simulink model "chapter2_5"  (R2022a)
settings: Solver=FixedStepAuto  StartTime=0.0  StopTime=8  FixedStep=3e-3  RelTol=1e-3
values from neighbouring files (symbols this model uses):
  A = 10    [Figure2_3.m]    (also in parameterLoadForchapter2_4.m = 0.0001; parameterLoadForchapter2_5.m = 0.0001)
  base = Mb*9.8/4+mw*9.8+0.5*9.8*Mv    (= 46305)    [parameterLoadForchapter2_5.m]
  d = 2.6    [parameterLoadForchapter2_4.m]    (also in parameterLoadForchapter2_5.m)
  I = 3055e-8    (= 3.055e-05)    [parameterLoadForchapter2_5.m]
  Jv = 3044    [parameterLoadForchapter2_4.m]    (also in parameterLoadForchapter2_5.m)
  kf = 6.67e7    (= 66700000)    [parameterLoadForchapter2_5.m]
  Kv = 1.5e6    (= 1500000)    [parameterLoadForchapter2_4.m]    (also in parameterLoadForchapter2_5.m)
  L = 15.6    [parameterLoadForchapter2_4.m]    (also in parameterLoadForchapter2_5.m = 210)
  Ln = LNs(i)    [Mchapter2_4.m]    (also in Mchapter2_5.m = LN_set(i))
  m = 7800*(7684e-6)    (= 59.9352)    [parameterLoadForchapter2_5.m]
  Mv = 4060    [parameterLoadForchapter2_4.m]    (also in parameterLoadForchapter2_5.m = 2030)
  mw = 1670    [parameterLoadForchapter2_4.m]    (also in parameterLoadForchapter2_5.m = 835)
  N1 = 0    [Mchapter2_5.m]
  N2 = 0    [Mchapter2_5.m]
  position = 0.5*L    (= 105)    [parameterLoadForchapter2_5.m]
  r = 0.42    [parameterLoadForchapter2_4.m]    (also in parameterLoadForchapter2_5.m)
  R = 400    [Figure2_3.m]    (also in parameterLoadForchapter2_4.m = 0.42; parameterLoadForchapter2_5.m = 0.42)
  v = vkmh/3.6    (= 13.8888888889)    [parameterLoadForchapter2_4.m]    (also in parameterLoadForchapter2_5.m = 27.7777777778)
  vkmh = 50    [parameterLoadForchapter2_4.m]    (also in parameterLoadForchapter2_5.m = 100)
(part 26 of 157)

== system chapter2_5/Subsystem5/Subsystem3/Subsystem2/Subsystem2 ==
  index : Inport
  F : Inport  Port=2
  Add : Sum
  Constant1 : Constant  Value=2
  Constant3 : Constant
  Demux : Demux  Outputs=2
  Subsystem : Subsystem
  Subsystem1 : Subsystem
  Subsystem2 : Subsystem
  Subsystem3 : Subsystem
  Out1 : Outport
  wires:
  Add/1 -> Out1/1
  Constant1/1 -> Subsystem1/2(k)
  Subsystem1/1(Out1) -> Subsystem2/1(uxt1)
  Subsystem1/2(tk) -> Subsystem2/2(tk)
  Subsystem/2(tk) -> Subsystem3/2(tk)
  Constant3/1 -> Subsystem/2(k)
  Subsystem/1(Out1) -> Subsystem3/1(uxt1)
  index/1 -> Subsystem/1(In1), Subsystem1/1(index)
  Subsystem3/1(U1) -> Add/1
  Subsystem2/1(U1) -> Add/2
  F/1 -> Demux/1
  Demux/1 -> Subsystem/3(In3)
  Demux/2 -> Subsystem1/3(F)

== system chapter2_5/Subsystem5/Subsystem3/Subsystem2/Subsystem21 ==
  index : Inport
  F : Inport  Port=2
  Add : Sum
  Constant1 : Constant  Value=2
  Constant3 : Constant
  Demux : Demux  Outputs=2
  Subsystem : Subsystem
  Subsystem1 : Subsystem
  Subsystem2 : Subsystem
  Subsystem3 : Subsystem
  Out1 : Outport
  wires:
  Add/1 -> Out1/1
  Constant1/1 -> Subsystem1/2(k)
  Subsystem1/1(Out1) -> Subsystem2/1(uxt1)
  Subsystem1/2(tk) -> Subsystem2/2(tk)
  Subsystem/2(tk) -> Subsystem3/2(tk)
  Constant3/1 -> Subsystem/2(k)
  Subsystem/1(Out1) -> Subsystem3/1(uxt1)
  index/1 -> Subsystem/1(In1), Subsystem1/1(index)
  Subsystem3/1(U1) -> Add/1
  Subsystem2/1(U1) -> Add/2
  F/1 -> Demux/1
  Demux/1 -> Subsystem/3(In3)
  Demux/2 -> Subsystem1/3(F)

== system chapter2_5/Subsystem5/Subsystem3/Subsystem2/Subsystem3 ==
  index : Inport
  F : Inport  Port=2
  Add : Sum
  Constant1 : Constant  Value=2
  Constant3 : Constant
  Demux : Demux  Outputs=2
  Subsystem : Subsystem
  Subsystem1 : Subsystem
  Subsystem2 : Subsystem
  Subsystem3 : Subsystem
  Out1 : Outport
  wires:
  Demux/2 -> Subsystem1/3(F)
  Demux/1 -> Subsystem/3(In3)
  F/1 -> Demux/1
  Subsystem2/1(U1) -> Add/2
  Subsystem3/1(U1) -> Add/1
  index/1 -> Subsystem1/1(index), Subsystem/1(In1)
  Subsystem/1(Out1) -> Subsystem3/1(uxt1)
  Constant3/1 -> Subsystem/2(k)
  Subsystem/2(tk) -> Subsystem3/2(tk)
  Subsystem1/2(tk) -> Subsystem2/2(tk)
  Subsystem1/1(Out1) -> Subsystem2/1(uxt1)
  Constant1/1 -> Subsystem1/2(k)
  Add/1 -> Out1/1

== system chapter2_5/Subsystem5/Subsystem3/Subsystem2/Subsystem4 ==
  index : Inport
  F : Inport  Port=2
  Add : Sum
  Constant1 : Constant  Value=2
  Constant3 : Constant
  Demux : Demux  Outputs=2
  Subsystem : Subsystem
  Subsystem1 : Subsystem
  Subsystem2 : Subsystem
  Subsystem3 : Subsystem
  Out1 : Outport
  wires:
  Add/1 -> Out1/1
  Constant1/1 -> Subsystem1/2(k)
  Subsystem1/1(Out1) -> Subsystem2/1(uxt1)
  Subsystem1/2(tk) -> Subsystem2/2(tk)
  Subsystem/2(tk) -> Subsystem3/2(tk)
  Constant3/1 -> Subsystem/2(k)
  Subsystem/1(Out1) -> Subsystem3/1(uxt1)
  index/1 -> Subsystem/1(In1), Subsystem1/1(index)
  Subsystem3/1(U1) -> Add/1
  Subsystem2/1(U1) -> Add/2
  F/1 -> Demux/1
  Demux/1 -> Subsystem/3(In3)
  Demux/2 -> Subsystem1/3(F)

== system chapter2_5/Subsystem5/Subsystem3/Subsystem2/Subsystem5 ==
  index : Inport
  F : Inport  Port=2
  Add : Sum
  Constant1 : Constant  Value=2
  Constant3 : Constant
  Demux : Demux  Outputs=2
  Subsystem : Subsystem
  Subsystem1 : Subsystem
  Subsystem2 : Subsystem
  Subsystem3 : Subsystem
  Out1 : Outport
  wires:
  Demux/2 -> Subsystem1/3(F)
  Demux/1 -> Subsystem/3(In3)
  F/1 -> Demux/1
  Subsystem2/1(U1) -> Add/2
  Subsystem3/1(U1) -> Add/1
  index/1 -> Subsystem1/1(index), Subsystem/1(In1)
  Subsystem/1(Out1) -> Subsystem3/1(uxt1)
  Constant3/1 -> Subsystem/2(k)
  Subsystem/2(tk) -> Subsystem3/2(tk)
  Subsystem1/2(tk) -> Subsystem2/2(tk)
  Subsystem1/1(Out1) -> Subsystem2/1(uxt1)
  Constant1/1 -> Subsystem1/2(k)
  Add/1 -> Out1/1

== system chapter2_5/Subsystem5/Subsystem3/Subsystem2/Subsystem6 ==
  index : Inport
  F : Inport  Port=2
  Add : Sum
  Constant1 : Constant  Value=2
  Constant3 : Constant
  Demux : Demux  Outputs=2
  Subsystem : Subsystem
  Subsystem1 : Subsystem
  Subsystem2 : Subsystem
  Subsystem3 : Subsystem
  Out1 : Outport
  wires:
  Demux/2 -> Subsystem1/3(F)
  Demux/1 -> Subsystem/3(In3)
  F/1 -> Demux/1
  Subsystem2/1(U1) -> Add/2
  Subsystem3/1(U1) -> Add/1
  index/1 -> Subsystem1/1(index), Subsystem/1(In1)
  Subsystem/1(Out1) -> Subsystem3/1(uxt1)
  Constant3/1 -> Subsystem/2(k)
  Subsystem/2(tk) -> Subsystem3/2(tk)
  Subsystem1/2(tk) -> Subsystem2/2(tk)
  Subsystem1/1(Out1) -> Subsystem2/1(uxt1)
  Constant1/1 -> Subsystem1/2(k)
  Add/1 -> Out1/1

== system chapter2_5/Subsystem5/Subsystem3/Subsystem2/Subsystem7 ==
  index : Inport
  F : Inport  Port=2
  Add : Sum
  Constant1 : Constant  Value=2
  Constant3 : Constant
  Demux : Demux  Outputs=2
  Subsystem : Subsystem
  Subsystem1 : Subsystem
  Subsystem2 : Subsystem
  Subsystem3 : Subsystem
  Out1 : Outport
  wires:
  Add/1 -> Out1/1
  Constant1/1 -> Subsystem1/2(k)
  Subsystem1/1(Out1) -> Subsystem2/1(uxt1)
  Subsystem1/2(tk) -> Subsystem2/2(tk)
  Subsystem/2(tk) -> Subsystem3/2(tk)
  Constant3/1 -> Subsystem/2(k)
  Subsystem/1(Out1) -> Subsystem3/1(uxt1)
  index/1 -> Subsystem/1(In1), Subsystem1/1(index)
  Subsystem3/1(U1) -> Add/1
  Subsystem2/1(U1) -> Add/2
  F/1 -> Demux/1
  Demux/1 -> Subsystem/3(In3)
  Demux/2 -> Subsystem1/3(F)

== system chapter2_5/Subsystem5/Subsystem3/Subsystem2/Subsystem8 ==
  index : Inport
  F : Inport  Port=2
  Add : Sum
  Constant1 : Constant  Value=2
  Constant3 : Constant
  Demux : Demux  Outputs=2
  Subsystem : Subsystem
  Subsystem1 : Subsystem
  Subsystem2 : Subsystem
  Subsystem3 : Subsystem
  Out1 : Outport
  wires:
  Add/1 -> Out1/1
  Constant1/1 -> Subsystem1/2(k)
  Subsystem1/1(Out1) -> Subsystem2/1(uxt1)
  Subsystem1/2(tk) -> Subsystem2/2(tk)
  Subsystem/2(tk) -> Subsystem3/2(tk)
  Constant3/1 -> Subsystem/2(k)
  Subsystem/1(Out1) -> Subsystem3/1(uxt1)
  index/1 -> Subsystem/1(In1), Subsystem1/1(index)
  Subsystem3/1(U1) -> Add/1
  Subsystem2/1(U1) -> Add/2
  F/1 -> Demux/1
  Demux/1 -> Subsystem/3(In3)
  Demux/2 -> Subsystem1/3(F)

== system chapter2_5/Subsystem5/Subsystem3/Subsystem2/Subsystem9 ==
  index : Inport
  F : Inport  Port=2
  Add : Sum
  Constant1 : Constant  Value=2
  Constant3 : Constant
  Demux : Demux  Outputs=2
  Subsystem : Subsystem
  Subsystem1 : Subsystem
  Subsystem2 : Subsystem
  Subsystem3 : Subsystem
  Out1 : Outport
  wires:
  Demux/2 -> Subsystem1/3(F)
  Demux/1 -> Subsystem/3(In3)
  F/1 -> Demux/1
  Subsystem2/1(U1) -> Add/2
  Subsystem3/1(U1) -> Add/1
  index/1 -> Subsystem1/1(index), Subsystem/1(In1)
  Subsystem/1(Out1) -> Subsystem3/1(uxt1)
  Constant3/1 -> Subsystem/2(k)
  Subsystem/2(tk) -> Subsystem3/2(tk)
  Subsystem1/2(tk) -> Subsystem2/2(tk)
  Subsystem1/1(Out1) -> Subsystem2/1(uxt1)
  Constant1/1 -> Subsystem1/2(k)
  Add/1 -> Out1/1

== system chapter2_5/Subsystem5/Subsystem3/Subsystem3/Subsystem1 ==
  index : Inport
  F : Inport  Port=2
  Add : Sum
  Constant1 : Constant  Value=2
  Constant3 : Constant
  Demux : Demux  Outputs=2
  Subsystem : Subsystem
  Subsystem1 : Subsystem
  Subsystem2 : Subsystem
  Subsystem3 : Subsystem
  Out1 : Outport
  wires:
  Add/1 -> Out1/1
  Constant1/1 -> Subsystem1/2(k)
  Subsystem1/1(Out1) -> Subsystem2/1(uxt1)
  Subsystem1/2(tk) -> Subsystem2/2(tk)
  Subsystem/2(tk) -> Subsystem3/2(tk)
  Constant3/1 -> Subsystem/2(k)
  Subsystem/1(Out1) -> Subsystem3/1(uxt1)
  index/1 -> Subsystem/1(In1), Subsystem1/1(index)
  Subsystem3/1(U1) -> Add/1
  Subsystem2/1(U1) -> Add/2
  F/1 -> Demux/1
  Demux/1 -> Subsystem/3(In3)
  Demux/2 -> Subsystem1/3(F)

== system chapter2_5/Subsystem5/Subsystem3/Subsystem3/Subsystem10 ==
  index : Inport
  F : Inport  Port=2
  Add : Sum
  Constant1 : Constant  Value=2
  Constant3 : Constant
  Demux : Demux  Outputs=2
  Subsystem : Subsystem
  Subsystem1 : Subsystem
  Subsystem2 : Subsystem
  Subsystem3 : Subsystem
  Out1 : Outport
  wires:
  Add/1 -> Out1/1
  Constant1/1 -> Subsystem1/2(k)
  Subsystem1/1(Out1) -> Subsystem2/1(uxt1)
  Subsystem1/2(tk) -> Subsystem2/2(tk)
  Subsystem/2(tk) -> Subsystem3/2(tk)
  Constant3/1 -> Subsystem/2(k)
  Subsystem/1(Out1) -> Subsystem3/1(uxt1)
  index/1 -> Subsystem/1(In1), Subsystem1/1(index)
  Subsystem3/1(U1) -> Add/1
  Subsystem2/1(U1) -> Add/2
  F/1 -> Demux/1
  Demux/1 -> Subsystem/3(In3)
  Demux/2 -> Subsystem1/3(F)

== system chapter2_5/Subsystem5/Subsystem3/Subsystem3/Subsystem11 ==
  index : Inport
  F : Inport  Port=2
  Add : Sum
  Constant1 : Constant  Value=2
  Constant3 : Constant
  Demux : Demux  Outputs=2
  Subsystem : Subsystem
  Subsystem1 : Subsystem
  Subsystem2 : Subsystem
  Subsystem3 : Subsystem
  Out1 : Outport
  wires:
  Demux/2 -> Subsystem1/3(F)
  Demux/1 -> Subsystem/3(In3)
  F/1 -> Demux/1
  Subsystem2/1(U1) -> Add/2
  Subsystem3/1(U1) -> Add/1
  index/1 -> Subsystem1/1(index), Subsystem/1(In1)
  Subsystem/1(Out1) -> Subsystem3/1(uxt1)
  Constant3/1 -> Subsystem/2(k)
  Subsystem/2(tk) -> Subsystem3/2(tk)
  Subsystem1/2(tk) -> Subsystem2/2(tk)
  Subsystem1/1(Out1) -> Subsystem2/1(uxt1)
  Constant1/1 -> Subsystem1/2(k)
  Add/1 -> Out1/1

== system chapter2_5/Subsystem5/Subsystem3/Subsystem3/Subsystem12 ==
  index : Inport
  F : Inport  Port=2
  Add : Sum
  Constant1 : Constant  Value=2
  Constant3 : Constant
  Demux : Demux  Outputs=2
  Subsystem : Subsystem
  Subsystem1 : Subsystem
  Subsystem2 : Subsystem
  Subsystem3 : Subsystem
  Out1 : Outport
  wires:
  Add/1 -> Out1/1
  Constant1/1 -> Subsystem1/2(k)
  Subsystem1/1(Out1) -> Subsystem2/1(uxt1)
  Subsystem1/2(tk) -> Subsystem2/2(tk)
  Subsystem/2(tk) -> Subsystem3/2(tk)
  Constant3/1 -> Subsystem/2(k)
  Subsystem/1(Out1) -> Subsystem3/1(uxt1)
  index/1 -> Subsystem/1(In1), Subsystem1/1(index)
  Subsystem3/1(U1) -> Add/1
  Subsystem2/1(U1) -> Add/2
  F/1 -> Demux/1
  Demux/1 -> Subsystem/3(In3)
  Demux/2 -> Subsystem1/3(F)

== system chapter2_5/Subsystem5/Subsystem3/Subsystem3/Subsystem13 ==
  index : Inport
  F : Inport  Port=2
  Add : Sum
  Constant1 : Constant  Value=2
  Constant3 : Constant
  Demux : Demux  Outputs=2
  Subsystem : Subsystem
  Subsystem1 : Subsystem
  Subsystem2 : Subsystem
  Subsystem3 : Subsystem
  Out1 : Outport
  wires:
  Demux/2 -> Subsystem1/3(F)
  Demux/1 -> Subsystem/3(In3)
  F/1 -> Demux/1
  Subsystem2/1(U1) -> Add/2
  Subsystem3/1(U1) -> Add/1
  index/1 -> Subsystem1/1(index), Subsystem/1(In1)
  Subsystem/1(Out1) -> Subsystem3/1(uxt1)
  Constant3/1 -> Subsystem/2(k)
  Subsystem/2(tk) -> Subsystem3/2(tk)
  Subsystem1/2(tk) -> Subsystem2/2(tk)
  Subsystem1/1(Out1) -> Subsystem2/1(uxt1)
  Constant1/1 -> Subsystem1/2(k)
  Add/1 -> Out1/1

== system chapter2_5/Subsystem5/Subsystem3/Subsystem3/Subsystem14 ==
  index : Inport
  F : Inport  Port=2
  Add : Sum
  Constant1 : Constant  Value=2
  Constant3 : Constant
  Demux : Demux  Outputs=2
  Subsystem : Subsystem
  Subsystem1 : Subsystem
  Subsystem2 : Subsystem
  Subsystem3 : Subsystem
  Out1 : Outport
  wires:
  Demux/2 -> Subsystem1/3(F)
  Demux/1 -> Subsystem/3(In3)
  F/1 -> Demux/1
  Subsystem2/1(U1) -> Add/2
  Subsystem3/1(U1) -> Add/1
  index/1 -> Subsystem1/1(index), Subsystem/1(In1)
  Subsystem/1(Out1) -> Subsystem3/1(uxt1)
  Constant3/1 -> Subsystem/2(k)
  Subsystem/2(tk) -> Subsystem3/2(tk)
  Subsystem1/2(tk) -> Subsystem2/2(tk)
  Subsystem1/1(Out1) -> Subsystem2/1(uxt1)
  Constant1/1 -> Subsystem1/2(k)
  Add/1 -> Out1/1

== system chapter2_5/Subsystem5/Subsystem3/Subsystem3/Subsystem15 ==
  index : Inport
  F : Inport  Port=2
  Add : Sum
  Constant1 : Constant  Value=2
  Constant3 : Constant
  Demux : Demux  Outputs=2
  Subsystem : Subsystem
  Subsystem1 : Subsystem
  Subsystem2 : Subsystem
  Subsystem3 : Subsystem
  Out1 : Outport
  wires:
  Add/1 -> Out1/1
  Constant1/1 -> Subsystem1/2(k)
  Subsystem1/1(Out1) -> Subsystem2/1(uxt1)
  Subsystem1/2(tk) -> Subsystem2/2(tk)
  Subsystem/2(tk) -> Subsystem3/2(tk)
  Constant3/1 -> Subsystem/2(k)
  Subsystem/1(Out1) -> Subsystem3/1(uxt1)
  index/1 -> Subsystem/1(In1), Subsystem1/1(index)
  Subsystem3/1(U1) -> Add/1
  Subsystem2/1(U1) -> Add/2
  F/1 -> Demux/1
  Demux/1 -> Subsystem/3(In3)
  Demux/2 -> Subsystem1/3(F)

== system chapter2_5/Subsystem5/Subsystem3/Subsystem3/Subsystem16 ==
  index : Inport
  F : Inport  Port=2
  Add : Sum
  Constant1 : Constant  Value=2
  Constant3 : Constant
  Demux : Demux  Outputs=2
  Subsystem : Subsystem
  Subsystem1 : Subsystem
  Subsystem2 : Subsystem
  Subsystem3 : Subsystem
  Out1 : Outport
  wires:
  Demux/2 -> Subsystem1/3(F)
  Demux/1 -> Subsystem/3(In3)
  F/1 -> Demux/1
  Subsystem2/1(U1) -> Add/2
  Subsystem3/1(U1) -> Add/1
  index/1 -> Subsystem1/1(index), Subsystem/1(In1)
  Subsystem/1(Out1) -> Subsystem3/1(uxt1)
  Constant3/1 -> Subsystem/2(k)
  Subsystem/2(tk) -> Subsystem3/2(tk)
  Subsystem1/2(tk) -> Subsystem2/2(tk)
  Subsystem1/1(Out1) -> Subsystem2/1(uxt1)
  Constant1/1 -> Subsystem1/2(k)
  Add/1 -> Out1/1

== system chapter2_5/Subsystem5/Subsystem3/Subsystem3/Subsystem17 ==
  index : Inport
  F : Inport  Port=2
  Add : Sum
  Constant1 : Constant  Value=2
  Constant3 : Constant
  Demux : Demux  Outputs=2
  Subsystem : Subsystem
  Subsystem1 : Subsystem
  Subsystem2 : Subsystem
  Subsystem3 : Subsystem
  Out1 : Outport
  wires:
  Add/1 -> Out1/1
  Constant1/1 -> Subsystem1/2(k)
  Subsystem1/1(Out1) -> Subsystem2/1(uxt1)
  Subsystem1/2(tk) -> Subsystem2/2(tk)
  Subsystem/2(tk) -> Subsystem3/2(tk)
  Constant3/1 -> Subsystem/2(k)
  Subsystem/1(Out1) -> Subsystem3/1(uxt1)
  index/1 -> Subsystem/1(In1), Subsystem1/1(index)
  Subsystem3/1(U1) -> Add/1
  Subsystem2/1(U1) -> Add/2
  F/1 -> Demux/1
  Demux/1 -> Subsystem/3(In3)
  Demux/2 -> Subsystem1/3(F)

== system chapter2_5/Subsystem5/Subsystem3/Subsystem3/Subsystem18 ==
  index : Inport
  F : Inport  Port=2
  Add : Sum
  Constant1 : Constant  Value=2
  Constant3 : Constant
  Demux : Demux  Outputs=2
  Subsystem : Subsystem
  Subsystem1 : Subsystem
  Subsystem2 : Subsystem
  Subsystem3 : Subsystem
  Out1 : Outport
  wires:
  Add/1 -> Out1/1
  Constant1/1 -> Subsystem1/2(k)
  Subsystem1/1(Out1) -> Subsystem2/1(uxt1)
  Subsystem1/2(tk) -> Subsystem2/2(tk)
  Subsystem/2(tk) -> Subsystem3/2(tk)
  Constant3/1 -> Subsystem/2(k)
  Subsystem/1(Out1) -> Subsystem3/1(uxt1)
  index/1 -> Subsystem/1(In1), Subsystem1/1(index)
  Subsystem3/1(U1) -> Add/1
  Subsystem2/1(U1) -> Add/2
  F/1 -> Demux/1
  Demux/1 -> Subsystem/3(In3)
  Demux/2 -> Subsystem1/3(F)

== system chapter2_5/Subsystem5/Subsystem3/Subsystem3/Subsystem19 ==
  index : Inport
  F : Inport  Port=2
  Add : Sum
  Constant1 : Constant  Value=2
  Constant3 : Constant
  Demux : Demux  Outputs=2
  Subsystem : Subsystem
  Subsystem1 : Subsystem
  Subsystem2 : Subsystem
  Subsystem3 : Subsystem
  Out1 : Outport
  wires:
  Demux/2 -> Subsystem1/3(F)
  Demux/1 -> Subsystem/3(In3)
  F/1 -> Demux/1
  Subsystem2/1(U1) -> Add/2
  Subsystem3/1(U1) -> Add/1
  index/1 -> Subsystem1/1(index), Subsystem/1(In1)
  Subsystem/1(Out1) -> Subsystem3/1(uxt1)
  Constant3/1 -> Subsystem/2(k)
  Subsystem/2(tk) -> Subsystem3/2(tk)
  Subsystem1/2(tk) -> Subsystem2/2(tk)
  Subsystem1/1(Out1) -> Subsystem2/1(uxt1)
  Constant1/1 -> Subsystem1/2(k)
  Add/1 -> Out1/1

== system chapter2_5/Subsystem5/Subsystem3/Subsystem3/Subsystem2 ==
  index : Inport
  F : Inport  Port=2
  Add : Sum
  Constant1 : Constant  Value=2
  Constant3 : Constant
  Demux : Demux  Outputs=2
  Subsystem : Subsystem
  Subsystem1 : Subsystem
  Subsystem2 : Subsystem
  Subsystem3 : Subsystem
  Out1 : Outport
  wires:
  Demux/2 -> Subsystem1/3(F)
  Demux/1 -> Subsystem/3(In3)
  F/1 -> Demux/1
  Subsystem2/1(U1) -> Add/2
  Subsystem3/1(U1) -> Add/1
  index/1 -> Subsystem1/1(index), Subsystem/1(In1)
  Subsystem/1(Out1) -> Subsystem3/1(uxt1)
  Constant3/1 -> Subsystem/2(k)
  Subsystem/2(tk) -> Subsystem3/2(tk)
  Subsystem1/2(tk) -> Subsystem2/2(tk)
  Subsystem1/1(Out1) -> Subsystem2/1(uxt1)
  Constant1/1 -> Subsystem1/2(k)
  Add/1 -> Out1/1

== system chapter2_5/Subsystem5/Subsystem3/Subsystem3/Subsystem21 ==
  index : Inport
  F : Inport  Port=2
  Add : Sum
  Constant1 : Constant  Value=2
  Constant3 : Constant
  Demux : Demux  Outputs=2
  Subsystem : Subsystem
  Subsystem1 : Subsystem
  Subsystem2 : Subsystem
  Subsystem3 : Subsystem
  Out1 : Outport
  wires:
  Demux/2 -> Subsystem1/3(F)
  Demux/1 -> Subsystem/3(In3)
  F/1 -> Demux/1
  Subsystem2/1(U1) -> Add/2
  Subsystem3/1(U1) -> Add/1
  index/1 -> Subsystem1/1(index), Subsystem/1(In1)
  Subsystem/1(Out1) -> Subsystem3/1(uxt1)
  Constant3/1 -> Subsystem/2(k)
  Subsystem/2(tk) -> Subsystem3/2(tk)
  Subsystem1/2(tk) -> Subsystem2/2(tk)
  Subsystem1/1(Out1) -> Subsystem2/1(uxt1)
  Constant1/1 -> Subsystem1/2(k)
  Add/1 -> Out1/1

== system chapter2_5/Subsystem5/Subsystem3/Subsystem3/Subsystem3 ==
  index : Inport
  F : Inport  Port=2
  Add : Sum
  Constant1 : Constant  Value=2
  Constant3 : Constant
  Demux : Demux  Outputs=2
  Subsystem : Subsystem
  Subsystem1 : Subsystem
  Subsystem2 : Subsystem
  Subsystem3 : Subsystem
  Out1 : Outport
  wires:
  Add/1 -> Out1/1
  Constant1/1 -> Subsystem1/2(k)
  Subsystem1/1(Out1) -> Subsystem2/1(uxt1)
  Subsystem1/2(tk) -> Subsystem2/2(tk)
  Subsystem/2(tk) -> Subsystem3/2(tk)
  Constant3/1 -> Subsystem/2(k)
  Subsystem/1(Out1) -> Subsystem3/1(uxt1)
  index/1 -> Subsystem/1(In1), Subsystem1/1(index)
  Subsystem3/1(U1) -> Add/1
  Subsystem2/1(U1) -> Add/2
  F/1 -> Demux/1
  Demux/1 -> Subsystem/3(In3)
  Demux/2 -> Subsystem1/3(F)

== system chapter2_5/Subsystem5/Subsystem3/Subsystem3/Subsystem4 ==
  index : Inport
  F : Inport  Port=2
  Add : Sum
  Constant1 : Constant  Value=2
  Constant3 : Constant
  Demux : Demux  Outputs=2
  Subsystem : Subsystem
  Subsystem1 : Subsystem
  Subsystem2 : Subsystem
  Subsystem3 : Subsystem
  Out1 : Outport
  wires:
  Demux/2 -> Subsystem1/3(F)
  Demux/1 -> Subsystem/3(In3)
  F/1 -> Demux/1
  Subsystem2/1(U1) -> Add/2
  Subsystem3/1(U1) -> Add/1
  index/1 -> Subsystem1/1(index), Subsystem/1(In1)
  Subsystem/1(Out1) -> Subsystem3/1(uxt1)
  Constant3/1 -> Subsystem/2(k)
  Subsystem/2(tk) -> Subsystem3/2(tk)
  Subsystem1/2(tk) -> Subsystem2/2(tk)
  Subsystem1/1(Out1) -> Subsystem2/1(uxt1)
  Constant1/1 -> Subsystem1/2(k)
  Add/1 -> Out1/1

== system chapter2_5/Subsystem5/Subsystem3/Subsystem3/Subsystem5 ==
  index : Inport
  F : Inport  Port=2
  Add : Sum
  Constant1 : Constant  Value=2
  Constant3 : Constant
  Demux : Demux  Outputs=2
  Subsystem : Subsystem
  Subsystem1 : Subsystem
  Subsystem2 : Subsystem
  Subsystem3 : Subsystem
  Out1 : Outport
  wires:
  Add/1 -> Out1/1
  Constant1/1 -> Subsystem1/2(k)
  Subsystem1/1(Out1) -> Subsystem2/1(uxt1)
  Subsystem1/2(tk) -> Subsystem2/2(tk)
  Subsystem/2(tk) -> Subsystem3/2(tk)
  Constant3/1 -> Subsystem/2(k)
  Subsystem/1(Out1) -> Subsystem3/1(uxt1)
  index/1 -> Subsystem/1(In1), Subsystem1/1(index)
  Subsystem3/1(U1) -> Add/1
  Subsystem2/1(U1) -> Add/2
  F/1 -> Demux/1
  Demux/1 -> Subsystem/3(In3)
  Demux/2 -> Subsystem1/3(F)

== system chapter2_5/Subsystem5/Subsystem3/Subsystem3/Subsystem6 ==
  index : Inport
  F : Inport  Port=2
  Add : Sum
  Constant1 : Constant  Value=2
  Constant3 : Constant
  Demux : Demux  Outputs=2
  Subsystem : Subsystem
  Subsystem1 : Subsystem
  Subsystem2 : Subsystem
  Subsystem3 : Subsystem
  Out1 : Outport
  wires:
  Add/1 -> Out1/1
  Constant1/1 -> Subsystem1/2(k)
  Subsystem1/1(Out1) -> Subsystem2/1(uxt1)
  Subsystem1/2(tk) -> Subsystem2/2(tk)
  Subsystem/2(tk) -> Subsystem3/2(tk)
  Constant3/1 -> Subsystem/2(k)
  Subsystem/1(Out1) -> Subsystem3/1(uxt1)
  index/1 -> Subsystem/1(In1), Subsystem1/1(index)
  Subsystem3/1(U1) -> Add/1
  Subsystem2/1(U1) -> Add/2
  F/1 -> Demux/1
  Demux/1 -> Subsystem/3(In3)
  Demux/2 -> Subsystem1/3(F)

== system chapter2_5/Subsystem5/Subsystem3/Subsystem3/Subsystem7 ==
  index : Inport
  F : Inport  Port=2
  Add : Sum
  Constant1 : Constant  Value=2
  Constant3 : Constant
  Demux : Demux  Outputs=2
  Subsystem : Subsystem
  Subsystem1 : Subsystem
  Subsystem2 : Subsystem
  Subsystem3 : Subsystem
  Out1 : Outport
  wires:
  Demux/2 -> Subsystem1/3(F)
  Demux/1 -> Subsystem/3(In3)
  F/1 -> Demux/1
  Subsystem2/1(U1) -> Add/2
  Subsystem3/1(U1) -> Add/1
  index/1 -> Subsystem1/1(index), Subsystem/1(In1)
  Subsystem/1(Out1) -> Subsystem3/1(uxt1)
  Constant3/1 -> Subsystem/2(k)
  Subsystem/2(tk) -> Subsystem3/2(tk)
  Subsystem1/2(tk) -> Subsystem2/2(tk)
  Subsystem1/1(Out1) -> Subsystem2/1(uxt1)
  Constant1/1 -> Subsystem1/2(k)
  Add/1 -> Out1/1

== system chapter2_5/Subsystem5/Subsystem3/Subsystem3/Subsystem8 ==
  index : Inport
  F : Inport  Port=2
  Add : Sum
  Constant1 : Constant  Value=2
  Constant3 : Constant
  Demux : Demux  Outputs=2
  Subsystem : Subsystem
  Subsystem1 : Subsystem
  Subsystem2 : Subsystem
  Subsystem3 : Subsystem
  Out1 : Outport
  wires:
  Demux/2 -> Subsystem1/3(F)
  Demux/1 -> Subsystem/3(In3)
  F/1 -> Demux/1
  Subsystem2/1(U1) -> Add/2
  Subsystem3/1(U1) -> Add/1
  index/1 -> Subsystem1/1(index), Subsystem/1(In1)
  Subsystem/1(Out1) -> Subsystem3/1(uxt1)
  Constant3/1 -> Subsystem/2(k)
  Subsystem/2(tk) -> Subsystem3/2(tk)
  Subsystem1/2(tk) -> Subsystem2/2(tk)
  Subsystem1/1(Out1) -> Subsystem2/1(uxt1)
  Constant1/1 -> Subsystem1/2(k)
  Add/1 -> Out1/1

== system chapter2_5/Subsystem5/Subsystem3/Subsystem3/Subsystem9 ==
  index : Inport
  F : Inport  Port=2
  Add : Sum
  Constant1 : Constant  Value=2
  Constant3 : Constant
  Demux : Demux  Outputs=2
  Subsystem : Subsystem
  Subsystem1 : Subsystem
  Subsystem2 : Subsystem
  Subsystem3 : Subsystem
  Out1 : Outport
  wires:
  Add/1 -> Out1/1
  Constant1/1 -> Subsystem1/2(k)
  Subsystem1/1(Out1) -> Subsystem2/1(uxt1)
  Subsystem1/2(tk) -> Subsystem2/2(tk)
  Subsystem/2(tk) -> Subsystem3/2(tk)
  Constant3/1 -> Subsystem/2(k)
  Subsystem/1(Out1) -> Subsystem3/1(uxt1)
  index/1 -> Subsystem/1(In1), Subsystem1/1(index)
  Subsystem3/1(U1) -> Add/1
  Subsystem2/1(U1) -> Add/2
  F/1 -> Demux/1
  Demux/1 -> Subsystem/3(In3)
  Demux/2 -> Subsystem1/3(F)

== system chapter2_5/Subsystem5/Subsystem3/Subsystem4/Subsystem1 ==
  index : Inport
  F : Inport  Port=2
  Add : Sum
  Constant1 : Constant  Value=2
  Constant3 : Constant
  Demux : Demux  Outputs=2
  Subsystem : Subsystem
  Subsystem1 : Subsystem
  Subsystem2 : Subsystem
  Subsystem3 : Subsystem
  Out1 : Outport
  wires:
  Demux/2 -> Subsystem1/3(F)
  Demux/1 -> Subsystem/3(In3)
  F/1 -> Demux/1
  Subsystem2/1(U1) -> Add/2
  Subsystem3/1(U1) -> Add/1
  index/1 -> Subsystem1/1(index), Subsystem/1(In1)
  Subsystem/1(Out1) -> Subsystem3/1(uxt1)
  Constant3/1 -> Subsystem/2(k)
  Subsystem/2(tk) -> Subsystem3/2(tk)
  Subsystem1/2(tk) -> Subsystem2/2(tk)
  Subsystem1/1(Out1) -> Subsystem2/1(uxt1)
  Constant1/1 -> Subsystem1/2(k)
  Add/1 -> Out1/1

== system chapter2_5/Subsystem5/Subsystem3/Subsystem4/Subsystem10 ==
  index : Inport
  F : Inport  Port=2
  Add : Sum
  Constant1 : Constant  Value=2
  Constant3 : Constant
  Demux : Demux  Outputs=2
  Subsystem : Subsystem
  Subsystem1 : Subsystem
  Subsystem2 : Subsystem
  Subsystem3 : Subsystem
  Out1 : Outport
  wires:
  Demux/2 -> Subsystem1/3(F)
  Demux/1 -> Subsystem/3(In3)
  F/1 -> Demux/1
  Subsystem2/1(U1) -> Add/2
  Subsystem3/1(U1) -> Add/1
  index/1 -> Subsystem1/1(index), Subsystem/1(In1)
  Subsystem/1(Out1) -> Subsystem3/1(uxt1)
  Constant3/1 -> Subsystem/2(k)
  Subsystem/2(tk) -> Subsystem3/2(tk)
  Subsystem1/2(tk) -> Subsystem2/2(tk)
  Subsystem1/1(Out1) -> Subsystem2/1(uxt1)
  Constant1/1 -> Subsystem1/2(k)
  Add/1 -> Out1/1

== system chapter2_5/Subsystem5/Subsystem3/Subsystem4/Subsystem11 ==
  index : Inport
  F : Inport  Port=2
  Add : Sum
  Constant1 : Constant  Value=2
  Constant3 : Constant
  Demux : Demux  Outputs=2
  Subsystem : Subsystem
  Subsystem1 : Subsystem
  Subsystem2 : Subsystem
  Subsystem3 : Subsystem
  Out1 : Outport
  wires:
  Add/1 -> Out1/1
  Constant1/1 -> Subsystem1/2(k)
  Subsystem1/1(Out1) -> Subsystem2/1(uxt1)
  Subsystem1/2(tk) -> Subsystem2/2(tk)
  Subsystem/2(tk) -> Subsystem3/2(tk)
  Constant3/1 -> Subsystem/2(k)
  Subsystem/1(Out1) -> Subsystem3/1(uxt1)
  index/1 -> Subsystem/1(In1), Subsystem1/1(index)
  Subsystem3/1(U1) -> Add/1
  Subsystem2/1(U1) -> Add/2
  F/1 -> Demux/1
  Demux/1 -> Subsystem/3(In3)
  Demux/2 -> Subsystem1/3(F)

== system chapter2_5/Subsystem5/Subsystem3/Subsystem4/Subsystem12 ==
  index : Inport
  F : Inport  Port=2
  Add : Sum
  Constant1 : Constant  Value=2
  Constant3 : Constant
  Demux : Demux  Outputs=2
  Subsystem : Subsystem
  Subsystem1 : Subsystem
  Subsystem2 : Subsystem
  Subsystem3 : Subsystem
  Out1 : Outport
  wires:
  Demux/2 -> Subsystem1/3(F)
  Demux/1 -> Subsystem/3(In3)
  F/1 -> Demux/1
  Subsystem2/1(U1) -> Add/2
  Subsystem3/1(U1) -> Add/1
  index/1 -> Subsystem1/1(index), Subsystem/1(In1)
  Subsystem/1(Out1) -> Subsystem3/1(uxt1)
  Constant3/1 -> Subsystem/2(k)
  Subsystem/2(tk) -> Subsystem3/2(tk)
  Subsystem1/2(tk) -> Subsystem2/2(tk)
  Subsystem1/1(Out1) -> Subsystem2/1(uxt1)
  Constant1/1 -> Subsystem1/2(k)
  Add/1 -> Out1/1

== system chapter2_5/Subsystem5/Subsystem3/Subsystem4/Subsystem13 ==
  index : Inport
  F : Inport  Port=2
  Add : Sum
  Constant1 : Constant  Value=2
  Constant3 : Constant
  Demux : Demux  Outputs=2
  Subsystem : Subsystem
  Subsystem1 : Subsystem
  Subsystem2 : Subsystem
  Subsystem3 : Subsystem
  Out1 : Outport
  wires:
  Add/1 -> Out1/1
  Constant1/1 -> Subsystem1/2(k)
  Subsystem1/1(Out1) -> Subsystem2/1(uxt1)
  Subsystem1/2(tk) -> Subsystem2/2(tk)
  Subsystem/2(tk) -> Subsystem3/2(tk)
  Constant3/1 -> Subsystem/2(k)
  Subsystem/1(Out1) -> Subsystem3/1(uxt1)
  index/1 -> Subsystem/1(In1), Subsystem1/1(index)
  Subsystem3/1(U1) -> Add/1
  Subsystem2/1(U1) -> Add/2
  F/1 -> Demux/1
  Demux/1 -> Subsystem/3(In3)
  Demux/2 -> Subsystem1/3(F)

== system chapter2_5/Subsystem5/Subsystem3/Subsystem4/Subsystem14 ==
  index : Inport
  F : Inport  Port=2
  Add : Sum
  Constant1 : Constant  Value=2
  Constant3 : Constant
  Demux : Demux  Outputs=2
  Subsystem : Subsystem
  Subsystem1 : Subsystem
  Subsystem2 : Subsystem
  Subsystem3 : Subsystem
  Out1 : Outport
  wires:
  Add/1 -> Out1/1
  Constant1/1 -> Subsystem1/2(k)
  Subsystem1/1(Out1) -> Subsystem2/1(uxt1)
  Subsystem1/2(tk) -> Subsystem2/2(tk)
  Subsystem/2(tk) -> Subsystem3/2(tk)
  Constant3/1 -> Subsystem/2(k)
  Subsystem/1(Out1) -> Subsystem3/1(uxt1)
  index/1 -> Subsystem/1(In1), Subsystem1/1(index)
  Subsystem3/1(U1) -> Add/1
  Subsystem2/1(U1) -> Add/2
  F/1 -> Demux/1
  Demux/1 -> Subsystem/3(In3)
  Demux/2 -> Subsystem1/3(F)

== system chapter2_5/Subsystem5/Subsystem3/Subsystem4/Subsystem15 ==
  index : Inport
  F : Inport  Port=2
  Add : Sum
  Constant1 : Constant  Value=2
  Constant3 : Constant
  Demux : Demux  Outputs=2
  Subsystem : Subsystem
  Subsystem1 : Subsystem
  Subsystem2 : Subsystem
  Subsystem3 : Subsystem
  Out1 : Outport
  wires:
  Demux/2 -> Subsystem1/3(F)
  Demux/1 -> Subsystem/3(In3)
  F/1 -> Demux/1
  Subsystem2/1(U1) -> Add/2
  Subsystem3/1(U1) -> Add/1
  index/1 -> Subsystem1/1(index), Subsystem/1(In1)
  Subsystem/1(Out1) -> Subsystem3/1(uxt1)
  Constant3/1 -> Subsystem/2(k)
  Subsystem/2(tk) -> Subsystem3/2(tk)
  Subsystem1/2(tk) -> Subsystem2/2(tk)
  Subsystem1/1(Out1) -> Subsystem2/1(uxt1)
  Constant1/1 -> Subsystem1/2(k)
  Add/1 -> Out1/1

== system chapter2_5/Subsystem5/Subsystem3/Subsystem4/Subsystem16 ==
  index : Inport
  F : Inport  Port=2
  Add : Sum
  Constant1 : Constant  Value=2
  Constant3 : Constant
  Demux : Demux  Outputs=2
  Subsystem : Subsystem
  Subsystem1 : Subsystem
  Subsystem2 : Subsystem
  Subsystem3 : Subsystem
  Out1 : Outport
  wires:
  Add/1 -> Out1/1
  Constant1/1 -> Subsystem1/2(k)
  Subsystem1/1(Out1) -> Subsystem2/1(uxt1)
  Subsystem1/2(tk) -> Subsystem2/2(tk)
  Subsystem/2(tk) -> Subsystem3/2(tk)
  Constant3/1 -> Subsystem/2(k)
  Subsystem/1(Out1) -> Subsystem3/1(uxt1)
  index/1 -> Subsystem/1(In1), Subsystem1/1(index)
  Subsystem3/1(U1) -> Add/1
  Subsystem2/1(U1) -> Add/2
  F/1 -> Demux/1
  Demux/1 -> Subsystem/3(In3)
  Demux/2 -> Subsystem1/3(F)

== system chapter2_5/Subsystem5/Subsystem3/Subsystem4/Subsystem17 ==
  index : Inport
  F : Inport  Port=2
  Add : Sum
  Constant1 : Constant  Value=2
  Constant3 : Constant
  Demux : Demux  Outputs=2
  Subsystem : Subsystem
  Subsystem1 : Subsystem
  Subsystem2 : Subsystem
  Subsystem3 : Subsystem
  Out1 : Outport
  wires:
  Demux/2 -> Subsystem1/3(F)
  Demux/1 -> Subsystem/3(In3)
  F/1 -> Demux/1
  Subsystem2/1(U1) -> Add/2
  Subsystem3/1(U1) -> Add/1
  index/1 -> Subsystem1/1(index), Subsystem/1(In1)
  Subsystem/1(Out1) -> Subsystem3/1(uxt1)
  Constant3/1 -> Subsystem/2(k)
  Subsystem/2(tk) -> Subsystem3/2(tk)
  Subsystem1/2(tk) -> Subsystem2/2(tk)
  Subsystem1/1(Out1) -> Subsystem2/1(uxt1)
  Constant1/1 -> Subsystem1/2(k)
  Add/1 -> Out1/1

== system chapter2_5/Subsystem5/Subsystem3/Subsystem4/Subsystem18 ==
  index : Inport
  F : Inport  Port=2
  Add : Sum
  Constant1 : Constant  Value=2
  Constant3 : Constant
  Demux : Demux  Outputs=2
  Subsystem : Subsystem
  Subsystem1 : Subsystem
  Subsystem2 : Subsystem
  Subsystem3 : Subsystem
  Out1 : Outport
  wires:
  Demux/2 -> Subsystem1/3(F)
  Demux/1 -> Subsystem/3(In3)
  F/1 -> Demux/1
  Subsystem2/1(U1) -> Add/2
  Subsystem3/1(U1) -> Add/1
  index/1 -> Subsystem1/1(index), Subsystem/1(In1)
  Subsystem/1(Out1) -> Subsystem3/1(uxt1)
  Constant3/1 -> Subsystem/2(k)
  Subsystem/2(tk) -> Subsystem3/2(tk)
  Subsystem1/2(tk) -> Subsystem2/2(tk)
  Subsystem1/1(Out1) -> Subsystem2/1(uxt1)
  Constant1/1 -> Subsystem1/2(k)
  Add/1 -> Out1/1

== system chapter2_5/Subsystem5/Subsystem3/Subsystem4/Subsystem19 ==
  index : Inport
  F : Inport  Port=2
  Add : Sum
  Constant1 : Constant  Value=2
  Constant3 : Constant
  Demux : Demux  Outputs=2
  Subsystem : Subsystem
  Subsystem1 : Subsystem
  Subsystem2 : Subsystem
  Subsystem3 : Subsystem
  Out1 : Outport
  wires:
  Add/1 -> Out1/1
  Constant1/1 -> Subsystem1/2(k)
  Subsystem1/1(Out1) -> Subsystem2/1(uxt1)
  Subsystem1/2(tk) -> Subsystem2/2(tk)
  Subsystem/2(tk) -> Subsystem3/2(tk)
  Constant3/1 -> Subsystem/2(k)
  Subsystem/1(Out1) -> Subsystem3/1(uxt1)
  index/1 -> Subsystem/1(In1), Subsystem1/1(index)
  Subsystem3/1(U1) -> Add/1
  Subsystem2/1(U1) -> Add/2
  F/1 -> Demux/1
  Demux/1 -> Subsystem/3(In3)
  Demux/2 -> Subsystem1/3(F)

== system chapter2_5/Subsystem5/Subsystem3/Subsystem4/Subsystem2 ==
  index : Inport
  F : Inport  Port=2
  Add : Sum
  Constant1 : Constant  Value=2
  Constant3 : Constant
  Demux : Demux  Outputs=2
  Subsystem : Subsystem
  Subsystem1 : Subsystem
  Subsystem2 : Subsystem
  Subsystem3 : Subsystem
  Out1 : Outport
  wires:
  Add/1 -> Out1/1
  Constant1/1 -> Subsystem1/2(k)
  Subsystem1/1(Out1) -> Subsystem2/1(uxt1)
  Subsystem1/2(tk) -> Subsystem2/2(tk)
  Subsystem/2(tk) -> Subsystem3/2(tk)
  Constant3/1 -> Subsystem/2(k)
  Subsystem/1(Out1) -> Subsystem3/1(uxt1)
  index/1 -> Subsystem/1(In1), Subsystem1/1(index)
  Subsystem3/1(U1) -> Add/1
  Subsystem2/1(U1) -> Add/2
  F/1 -> Demux/1
  Demux/1 -> Subsystem/3(In3)
  Demux/2 -> Subsystem1/3(F)

== system chapter2_5/Subsystem5/Subsystem3/Subsystem4/Subsystem21 ==
  index : Inport
  F : Inport  Port=2
  Add : Sum
  Constant1 : Constant  Value=2
  Constant3 : Constant
  Demux : Demux  Outputs=2
  Subsystem : Subsystem
  Subsystem1 : Subsystem
  Subsystem2 : Subsystem
  Subsystem3 : Subsystem
  Out1 : Outport
  wires:
  Add/1 -> Out1/1
  Constant1/1 -> Subsystem1/2(k)
  Subsystem1/1(Out1) -> Subsystem2/1(uxt1)
  Subsystem1/2(tk) -> Subsystem2/2(tk)
  Subsystem/2(tk) -> Subsystem3/2(tk)
  Constant3/1 -> Subsystem/2(k)
  Subsystem/1(Out1) -> Subsystem3/1(uxt1)
  index/1 -> Subsystem/1(In1), Subsystem1/1(index)
  Subsystem3/1(U1) -> Add/1
  Subsystem2/1(U1) -> Add/2
  F/1 -> Demux/1
  Demux/1 -> Subsystem/3(In3)
  Demux/2 -> Subsystem1/3(F)

== system chapter2_5/Subsystem5/Subsystem3/Subsystem4/Subsystem3 ==
  index : Inport
  F : Inport  Port=2
  Add : Sum
  Constant1 : Constant  Value=2
  Constant3 : Constant
  Demux : Demux  Outputs=2
  Subsystem : Subsystem
  Subsystem1 : Subsystem
  Subsystem2 : Subsystem
  Subsystem3 : Subsystem
  Out1 : Outport
  wires:
  Demux/2 -> Subsystem1/3(F)
  Demux/1 -> Subsystem/3(In3)
  F/1 -> Demux/1
  Subsystem2/1(U1) -> Add/2
  Subsystem3/1(U1) -> Add/1
  index/1 -> Subsystem1/1(index), Subsystem/1(In1)
  Subsystem/1(Out1) -> Subsystem3/1(uxt1)
  Constant3/1 -> Subsystem/2(k)
  Subsystem/2(tk) -> Subsystem3/2(tk)
  Subsystem1/2(tk) -> Subsystem2/2(tk)
  Subsystem1/1(Out1) -> Subsystem2/1(uxt1)
  Constant1/1 -> Subsystem1/2(k)
  Add/1 -> Out1/1

== system chapter2_5/Subsystem5/Subsystem3/Subsystem4/Subsystem4 ==
  index : Inport
  F : Inport  Port=2
  Add : Sum
  Constant1 : Constant  Value=2
  Constant3 : Constant
  Demux : Demux  Outputs=2
  Subsystem : Subsystem
  Subsystem1 : Subsystem
  Subsystem2 : Subsystem
  Subsystem3 : Subsystem
  Out1 : Outport
  wires:
  Add/1 -> Out1/1
  Constant1/1 -> Subsystem1/2(k)
  Subsystem1/1(Out1) -> Subsystem2/1(uxt1)
  Subsystem1/2(tk) -> Subsystem2/2(tk)
  Subsystem/2(tk) -> Subsystem3/2(tk)
  Constant3/1 -> Subsystem/2(k)
  Subsystem/1(Out1) -> Subsystem3/1(uxt1)
  index/1 -> Subsystem/1(In1), Subsystem1/1(index)
  Subsystem3/1(U1) -> Add/1
  Subsystem2/1(U1) -> Add/2
  F/1 -> Demux/1
  Demux/1 -> Subsystem/3(In3)
  Demux/2 -> Subsystem1/3(F)

== system chapter2_5/Subsystem5/Subsystem3/Subsystem4/Subsystem5 ==
  index : Inport
  F : Inport  Port=2
  Add : Sum
  Constant1 : Constant  Value=2
  Constant3 : Constant
  Demux : Demux  Outputs=2
  Subsystem : Subsystem
  Subsystem1 : Subsystem
  Subsystem2 : Subsystem
  Subsystem3 : Subsystem
  Out1 : Outport
  wires:
  Demux/2 -> Subsystem1/3(F)
  Demux/1 -> Subsystem/3(In3)
  F/1 -> Demux/1
  Subsystem2/1(U1) -> Add/2
  Subsystem3/1(U1) -> Add/1
  index/1 -> Subsystem1/1(index), Subsystem/1(In1)
  Subsystem/1(Out1) -> Subsystem3/1(uxt1)
  Constant3/1 -> Subsystem/2(k)
  Subsystem/2(tk) -> Subsystem3/2(tk)
  Subsystem1/2(tk) -> Subsystem2/2(tk)
  Subsystem1/1(Out1) -> Subsystem2/1(uxt1)
  Constant1/1 -> Subsystem1/2(k)
  Add/1 -> Out1/1

== system chapter2_5/Subsystem5/Subsystem3/Subsystem4/Subsystem6 ==
  index : Inport
  F : Inport  Port=2
  Add : Sum
  Constant1 : Constant  Value=2
  Constant3 : Constant
  Demux : Demux  Outputs=2
  Subsystem : Subsystem
  Subsystem1 : Subsystem
  Subsystem2 : Subsystem
  Subsystem3 : Subsystem
  Out1 : Outport
  wires:
  Demux/2 -> Subsystem1/3(F)
  Demux/1 -> Subsystem/3(In3)
  F/1 -> Demux/1
  Subsystem2/1(U1) -> Add/2
  Subsystem3/1(U1) -> Add/1
  index/1 -> Subsystem1/1(index), Subsystem/1(In1)
  Subsystem/1(Out1) -> Subsystem3/1(uxt1)
  Constant3/1 -> Subsystem/2(k)
  Subsystem/2(tk) -> Subsystem3/2(tk)
  Subsystem1/2(tk) -> Subsystem2/2(tk)
  Subsystem1/1(Out1) -> Subsystem2/1(uxt1)
  Constant1/1 -> Subsystem1/2(k)
  Add/1 -> Out1/1

== system chapter2_5/Subsystem5/Subsystem3/Subsystem4/Subsystem7 ==
  index : Inport
  F : Inport  Port=2
  Add : Sum
  Constant1 : Constant  Value=2
  Constant3 : Constant
  Demux : Demux  Outputs=2
  Subsystem : Subsystem
  Subsystem1 : Subsystem
  Subsystem2 : Subsystem
  Subsystem3 : Subsystem
  Out1 : Outport
  wires:
  Add/1 -> Out1/1
  Constant1/1 -> Subsystem1/2(k)
  Subsystem1/1(Out1) -> Subsystem2/1(uxt1)
  Subsystem1/2(tk) -> Subsystem2/2(tk)
  Subsystem/2(tk) -> Subsystem3/2(tk)
  Constant3/1 -> Subsystem/2(k)
  Subsystem/1(Out1) -> Subsystem3/1(uxt1)
  index/1 -> Subsystem/1(In1), Subsystem1/1(index)
  Subsystem3/1(U1) -> Add/1
  Subsystem2/1(U1) -> Add/2
  F/1 -> Demux/1
  Demux/1 -> Subsystem/3(In3)
  Demux/2 -> Subsystem1/3(F)

== system chapter2_5/Subsystem5/Subsystem3/Subsystem4/Subsystem8 ==
  index : Inport
  F : Inport  Port=2
  Add : Sum
  Constant1 : Constant  Value=2
  Constant3 : Constant
  Demux : Demux  Outputs=2
  Subsystem : Subsystem
  Subsystem1 : Subsystem
  Subsystem2 : Subsystem
  Subsystem3 : Subsystem
  Out1 : Outport
  wires:
  Add/1 -> Out1/1
  Constant1/1 -> Subsystem1/2(k)
  Subsystem1/1(Out1) -> Subsystem2/1(uxt1)
  Subsystem1/2(tk) -> Subsystem2/2(tk)
  Subsystem/2(tk) -> Subsystem3/2(tk)
  Constant3/1 -> Subsystem/2(k)
  Subsystem/1(Out1) -> Subsystem3/1(uxt1)
  index/1 -> Subsystem/1(In1), Subsystem1/1(index)
  Subsystem3/1(U1) -> Add/1
  Subsystem2/1(U1) -> Add/2
  F/1 -> Demux/1
  Demux/1 -> Subsystem/3(In3)
  Demux/2 -> Subsystem1/3(F)

== system chapter2_5/Subsystem5/Subsystem3/Subsystem4/Subsystem9 ==
  index : Inport
  F : Inport  Port=2
  Add : Sum
  Constant1 : Constant  Value=2
  Constant3 : Constant
  Demux : Demux  Outputs=2
  Subsystem : Subsystem
  Subsystem1 : Subsystem
  Subsystem2 : Subsystem
  Subsystem3 : Subsystem
  Out1 : Outport
  wires:
  Demux/2 -> Subsystem1/3(F)
  Demux/1 -> Subsystem/3(In3)
  F/1 -> Demux/1
  Subsystem2/1(U1) -> Add/2
  Subsystem3/1(U1) -> Add/1
  index/1 -> Subsystem1/1(index), Subsystem/1(In1)
  Subsystem/1(Out1) -> Subsystem3/1(uxt1)
  Constant3/1 -> Subsystem/2(k)
  Subsystem/2(tk) -> Subsystem3/2(tk)
  Subsystem1/2(tk) -> Subsystem2/2(tk)
  Subsystem1/1(Out1) -> Subsystem2/1(uxt1)
  Constant1/1 -> Subsystem1/2(k)
  Add/1 -> Out1/1

== system chapter2_5/Subsystem5/Subsystem3/Subsystem5/Subsystem1 ==
  index : Inport
  F : Inport  Port=2
  Add : Sum
  Constant1 : Constant  Value=2
  Constant3 : Constant
  Demux : Demux  Outputs=2
  Subsystem : Subsystem
  Subsystem1 : Subsystem
  Subsystem2 : Subsystem
  Subsystem3 : Subsystem
  Out1 : Outport
  wires:
  Demux/2 -> Subsystem1/3(F)
  Demux/1 -> Subsystem/3(In3)
  F/1 -> Demux/1
  Subsystem2/1(U1) -> Add/2
  Subsystem3/1(U1) -> Add/1
  index/1 -> Subsystem1/1(index), Subsystem/1(In1)
  Subsystem/1(Out1) -> Subsystem3/1(uxt1)
  Constant3/1 -> Subsystem/2(k)
  Subsystem/2(tk) -> Subsystem3/2(tk)
  Subsystem1/2(tk) -> Subsystem2/2(tk)
  Subsystem1/1(Out1) -> Subsystem2/1(uxt1)
  Constant1/1 -> Subsystem1/2(k)
  Add/1 -> Out1/1

== system chapter2_5/Subsystem5/Subsystem3/Subsystem5/Subsystem10 ==
  index : Inport
  F : Inport  Port=2
  Add : Sum
  Constant1 : Constant  Value=2
  Constant3 : Constant
  Demux : Demux  Outputs=2
  Subsystem : Subsystem
  Subsystem1 : Subsystem
  Subsystem2 : Subsystem
  Subsystem3 : Subsystem
  Out1 : Outport
  wires:
  Demux/2 -> Subsystem1/3(F)
  Demux/1 -> Subsystem/3(In3)
  F/1 -> Demux/1
  Subsystem2/1(U1) -> Add/2
  Subsystem3/1(U1) -> Add/1
  index/1 -> Subsystem1/1(index), Subsystem/1(In1)
  Subsystem/1(Out1) -> Subsystem3/1(uxt1)
  Constant3/1 -> Subsystem/2(k)
  Subsystem/2(tk) -> Subsystem3/2(tk)
  Subsystem1/2(tk) -> Subsystem2/2(tk)
  Subsystem1/1(Out1) -> Subsystem2/1(uxt1)
  Constant1/1 -> Subsystem1/2(k)
  Add/1 -> Out1/1

== system chapter2_5/Subsystem5/Subsystem3/Subsystem5/Subsystem11 ==
  index : Inport
  F : Inport  Port=2
  Add : Sum
  Constant1 : Constant  Value=2
  Constant3 : Constant
  Demux : Demux  Outputs=2
  Subsystem : Subsystem
  Subsystem1 : Subsystem
  Subsystem2 : Subsystem
  Subsystem3 : Subsystem
  Out1 : Outport
  wires:
  Add/1 -> Out1/1
  Constant1/1 -> Subsystem1/2(k)
  Subsystem1/1(Out1) -> Subsystem2/1(uxt1)
  Subsystem1/2(tk) -> Subsystem2/2(tk)
  Subsystem/2(tk) -> Subsystem3/2(tk)
  Constant3/1 -> Subsystem/2(k)
  Subsystem/1(Out1) -> Subsystem3/1(uxt1)
  index/1 -> Subsystem/1(In1), Subsystem1/1(index)
  Subsystem3/1(U1) -> Add/1
  Subsystem2/1(U1) -> Add/2
  F/1 -> Demux/1
  Demux/1 -> Subsystem/3(In3)
  Demux/2 -> Subsystem1/3(F)

== system chapter2_5/Subsystem5/Subsystem3/Subsystem5/Subsystem12 ==
  index : Inport
  F : Inport  Port=2
  Add : Sum
  Constant1 : Constant  Value=2
  Constant3 : Constant
  Demux : Demux  Outputs=2
  Subsystem : Subsystem
  Subsystem1 : Subsystem
  Subsystem2 : Subsystem
  Subsystem3 : Subsystem
  Out1 : Outport
  wires:
  Demux/2 -> Subsystem1/3(F)
  Demux/1 -> Subsystem/3(In3)
  F/1 -> Demux/1
  Subsystem2/1(U1) -> Add/2
  Subsystem3/1(U1) -> Add/1
  index/1 -> Subsystem1/1(index), Subsystem/1(In1)
  Subsystem/1(Out1) -> Subsystem3/1(uxt1)
  Constant3/1 -> Subsystem/2(k)
  Subsystem/2(tk) -> Subsystem3/2(tk)
  Subsystem1/2(tk) -> Subsystem2/2(tk)
  Subsystem1/1(Out1) -> Subsystem2/1(uxt1)
  Constant1/1 -> Subsystem1/2(k)
  Add/1 -> Out1/1

== system chapter2_5/Subsystem5/Subsystem3/Subsystem5/Subsystem13 ==
  index : Inport
  F : Inport  Port=2
  Add : Sum
  Constant1 : Constant  Value=2
  Constant3 : Constant
  Demux : Demux  Outputs=2
  Subsystem : Subsystem
  Subsystem1 : Subsystem
  Subsystem2 : Subsystem
  Subsystem3 : Subsystem
  Out1 : Outport
  wires:
  Add/1 -> Out1/1
  Constant1/1 -> Subsystem1/2(k)
  Subsystem1/1(Out1) -> Subsystem2/1(uxt1)
  Subsystem1/2(tk) -> Subsystem2/2(tk)
  Subsystem/2(tk) -> Subsystem3/2(tk)
  Constant3/1 -> Subsystem/2(k)
  Subsystem/1(Out1) -> Subsystem3/1(uxt1)
  index/1 -> Subsystem/1(In1), Subsystem1/1(index)
  Subsystem3/1(U1) -> Add/1
  Subsystem2/1(U1) -> Add/2
  F/1 -> Demux/1
  Demux/1 -> Subsystem/3(In3)
  Demux/2 -> Subsystem1/3(F)

== system chapter2_5/Subsystem5/Subsystem3/Subsystem5/Subsystem14 ==
  index : Inport
  F : Inport  Port=2
  Add : Sum
  Constant1 : Constant  Value=2
  Constant3 : Constant
  Demux : Demux  Outputs=2
  Subsystem : Subsystem
  Subsystem1 : Subsystem
  Subsystem2 : Subsystem
  Subsystem3 : Subsystem
  Out1 : Outport
  wires:
  Add/1 -> Out1/1
  Constant1/1 -> Subsystem1/2(k)
  Subsystem1/1(Out1) -> Subsystem2/1(uxt1)
  Subsystem1/2(tk) -> Subsystem2/2(tk)
  Subsystem/2(tk) -> Subsystem3/2(tk)
  Constant3/1 -> Subsystem/2(k)
  Subsystem/1(Out1) -> Subsystem3/1(uxt1)
  index/1 -> Subsystem/1(In1), Subsystem1/1(index)
  Subsystem3/1(U1) -> Add/1
  Subsystem2/1(U1) -> Add/2
  F/1 -> Demux/1
  Demux/1 -> Subsystem/3(In3)
  Demux/2 -> Subsystem1/3(F)

== system chapter2_5/Subsystem5/Subsystem3/Subsystem5/Subsystem15 ==
  index : Inport
  F : Inport  Port=2
  Add : Sum
  Constant1 : Constant  Value=2
  Constant3 : Constant
  Demux : Demux  Outputs=2
  Subsystem : Subsystem
  Subsystem1 : Subsystem
  Subsystem2 : Subsystem
  Subsystem3 : Subsystem
  Out1 : Outport
  wires:
  Demux/2 -> Subsystem1/3(F)
  Demux/1 -> Subsystem/3(In3)
  F/1 -> Demux/1
  Subsystem2/1(U1) -> Add/2
  Subsystem3/1(U1) -> Add/1
  index/1 -> Subsystem1/1(index), Subsystem/1(In1)
  Subsystem/1(Out1) -> Subsystem3/1(uxt1)
  Constant3/1 -> Subsystem/2(k)
  Subsystem/2(tk) -> Subsystem3/2(tk)
  Subsystem1/2(tk) -> Subsystem2/2(tk)
  Subsystem1/1(Out1) -> Subsystem2/1(uxt1)
  Constant1/1 -> Subsystem1/2(k)
  Add/1 -> Out1/1

== system chapter2_5/Subsystem5/Subsystem3/Subsystem5/Subsystem16 ==
  index : Inport
  F : Inport  Port=2
  Add : Sum
  Constant1 : Constant  Value=2
  Constant3 : Constant
  Demux : Demux  Outputs=2
  Subsystem : Subsystem
  Subsystem1 : Subsystem
  Subsystem2 : Subsystem
  Subsystem3 : Subsystem
  Out1 : Outport
  wires:
  Add/1 -> Out1/1
  Constant1/1 -> Subsystem1/2(k)
  Subsystem1/1(Out1) -> Subsystem2/1(uxt1)
  Subsystem1/2(tk) -> Subsystem2/2(tk)
  Subsystem/2(tk) -> Subsystem3/2(tk)
  Constant3/1 -> Subsystem/2(k)
  Subsystem/1(Out1) -> Subsystem3/1(uxt1)
  index/1 -> Subsystem/1(In1), Subsystem1/1(index)
  Subsystem3/1(U1) -> Add/1
  Subsystem2/1(U1) -> Add/2
  F/1 -> Demux/1
  Demux/1 -> Subsystem/3(In3)
  Demux/2 -> Subsystem1/3(F)

== system chapter2_5/Subsystem5/Subsystem3/Subsystem5/Subsystem17 ==
  index : Inport
  F : Inport  Port=2
  Add : Sum
  Constant1 : Constant  Value=2
  Constant3 : Constant
  Demux : Demux  Outputs=2
  Subsystem : Subsystem
  Subsystem1 : Subsystem
  Subsystem2 : Subsystem
  Subsystem3 : Subsystem
  Out1 : Outport
  wires:
  Demux/2 -> Subsystem1/3(F)
  Demux/1 -> Subsystem/3(In3)
  F/1 -> Demux/1
  Subsystem2/1(U1) -> Add/2
  Subsystem3/1(U1) -> Add/1
  index/1 -> Subsystem1/1(index), Subsystem/1(In1)
  Subsystem/1(Out1) -> Subsystem3/1(uxt1)
  Constant3/1 -> Subsystem/2(k)
  Subsystem/2(tk) -> Subsystem3/2(tk)
  Subsystem1/2(tk) -> Subsystem2/2(tk)
  Subsystem1/1(Out1) -> Subsystem2/1(uxt1)
  Constant1/1 -> Subsystem1/2(k)
  Add/1 -> Out1/1

== system chapter2_5/Subsystem5/Subsystem3/Subsystem5/Subsystem18 ==
  index : Inport
  F : Inport  Port=2
  Add : Sum
  Constant1 : Constant  Value=2
  Constant3 : Constant
  Demux : Demux  Outputs=2
  Subsystem : Subsystem
  Subsystem1 : Subsystem
  Subsystem2 : Subsystem
  Subsystem3 : Subsystem
  Out1 : Outport
  wires:
  Demux/2 -> Subsystem1/3(F)
  Demux/1 -> Subsystem/3(In3)
  F/1 -> Demux/1
  Subsystem2/1(U1) -> Add/2
  Subsystem3/1(U1) -> Add/1
  index/1 -> Subsystem1/1(index), Subsystem/1(In1)
  Subsystem/1(Out1) -> Subsystem3/1(uxt1)
  Constant3/1 -> Subsystem/2(k)
  Subsystem/2(tk) -> Subsystem3/2(tk)
  Subsystem1/2(tk) -> Subsystem2/2(tk)
  Subsystem1/1(Out1) -> Subsystem2/1(uxt1)
  Constant1/1 -> Subsystem1/2(k)
  Add/1 -> Out1/1

== system chapter2_5/Subsystem5/Subsystem3/Subsystem5/Subsystem19 ==
  index : Inport
  F : Inport  Port=2
  Add : Sum
  Constant1 : Constant  Value=2
  Constant3 : Constant
  Demux : Demux  Outputs=2
  Subsystem : Subsystem
  Subsystem1 : Subsystem
  Subsystem2 : Subsystem
  Subsystem3 : Subsystem
  Out1 : Outport
  wires:
  Add/1 -> Out1/1
  Constant1/1 -> Subsystem1/2(k)
  Subsystem1/1(Out1) -> Subsystem2/1(uxt1)
  Subsystem1/2(tk) -> Subsystem2/2(tk)
  Subsystem/2(tk) -> Subsystem3/2(tk)
  Constant3/1 -> Subsystem/2(k)
  Subsystem/1(Out1) -> Subsystem3/1(uxt1)
  index/1 -> Subsystem/1(In1), Subsystem1/1(index)
  Subsystem3/1(U1) -> Add/1
  Subsystem2/1(U1) -> Add/2
  F/1 -> Demux/1
  Demux/1 -> Subsystem/3(In3)
  Demux/2 -> Subsystem1/3(F)

== system chapter2_5/Subsystem5/Subsystem3/Subsystem5/Subsystem2 ==
  index : Inport
  F : Inport  Port=2
  Add : Sum
  Constant1 : Constant  Value=2
  Constant3 : Constant
  Demux : Demux  Outputs=2
  Subsystem : Subsystem
  Subsystem1 : Subsystem
  Subsystem2 : Subsystem
  Subsystem3 : Subsystem
  Out1 : Outport
  wires:
  Add/1 -> Out1/1
  Constant1/1 -> Subsystem1/2(k)
  Subsystem1/1(Out1) -> Subsystem2/1(uxt1)
  Subsystem1/2(tk) -> Subsystem2/2(tk)
  Subsystem/2(tk) -> Subsystem3/2(tk)
  Constant3/1 -> Subsystem/2(k)
  Subsystem/1(Out1) -> Subsystem3/1(uxt1)
  index/1 -> Subsystem/1(In1), Subsystem1/1(index)
  Subsystem3/1(U1) -> Add/1
  Subsystem2/1(U1) -> Add/2
  F/1 -> Demux/1
  Demux/1 -> Subsystem/3(In3)
  Demux/2 -> Subsystem1/3(F)

== system chapter2_5/Subsystem5/Subsystem3/Subsystem5/Subsystem21 ==
  index : Inport
  F : Inport  Port=2
  Add : Sum
  Constant1 : Constant  Value=2
  Constant3 : Constant
  Demux : Demux  Outputs=2
  Subsystem : Subsystem
  Subsystem1 : Subsystem
  Subsystem2 : Subsystem
  Subsystem3 : Subsystem
  Out1 : Outport
  wires:
  Add/1 -> Out1/1
  Constant1/1 -> Subsystem1/2(k)
  Subsystem1/1(Out1) -> Subsystem2/1(uxt1)
  Subsystem1/2(tk) -> Subsystem2/2(tk)
  Subsystem/2(tk) -> Subsystem3/2(tk)
  Constant3/1 -> Subsystem/2(k)
  Subsystem/1(Out1) -> Subsystem3/1(uxt1)
  index/1 -> Subsystem/1(In1), Subsystem1/1(index)
  Subsystem3/1(U1) -> Add/1
  Subsystem2/1(U1) -> Add/2
  F/1 -> Demux/1
  Demux/1 -> Subsystem/3(In3)
  Demux/2 -> Subsystem1/3(F)

== system chapter2_5/Subsystem5/Subsystem3/Subsystem5/Subsystem3 ==
  index : Inport
  F : Inport  Port=2
  Add : Sum
  Constant1 : Constant  Value=2
  Constant3 : Constant
  Demux : Demux  Outputs=2
  Subsystem : Subsystem
  Subsystem1 : Subsystem
  Subsystem2 : Subsystem
  Subsystem3 : Subsystem
  Out1 : Outport
  wires:
  Demux/2 -> Subsystem1/3(F)
  Demux/1 -> Subsystem/3(In3)
  F/1 -> Demux/1
  Subsystem2/1(U1) -> Add/2
  Subsystem3/1(U1) -> Add/1
  index/1 -> Subsystem1/1(index), Subsystem/1(In1)
  Subsystem/1(Out1) -> Subsystem3/1(uxt1)
  Constant3/1 -> Subsystem/2(k)
  Subsystem/2(tk) -> Subsystem3/2(tk)
  Subsystem1/2(tk) -> Subsystem2/2(tk)
  Subsystem1/1(Out1) -> Subsystem2/1(uxt1)
  Constant1/1 -> Subsystem1/2(k)
  Add/1 -> Out1/1
